simulink model "os_polyphase_synth_4096c"  (R2021a) (continued)
== system os_polyphase_synth_4096c/pfb_fir/pol1_in1_tap1 == (continued)
      | C+P>>17+(D-ACIN)*B
      | C+P>>17+(D-ACIN)*B+CARRYIN
      | C+P>>17+(D-ACIN)*BCIN
      | C+P>>17+(D-ACIN)*BCIN+CARRYIN
      | C+P>>17+(D-ACIN)+CARRYIN
      | C+P>>17+A
      | C+P>>17+A*A
      | C+P>>17+A*A+CARRYIN
      | C+P>>17+A*B
      | C+P>>17+A*B+CARRYIN
      | C+P>>17+A*BCIN
      | C+P>>17+A*BCIN+CARRYIN
      | C+P>>17+A+CARRYIN
      | C+P>>17+ACIN
      | C+P>>17+ACIN*ACIN
      | C+P>>17+ACIN*ACIN+CARRYIN
      | C+P>>17+ACIN*B
      | C+P>>17+ACIN*B+CARRYIN
      | C+P>>17+ACIN*BCIN
      | C+P>>17+ACIN*BCIN+CARRYIN
      | C+P>>17+ACIN+CARRYIN
      | C+P>>17+B*(A+D)
      | C+P>>17+B*(A+D)+CARRYIN
      | C+P>>17+B*(ACIN+D)
      | C+P>>17+B*(ACIN+D)+CARRYIN
      | C+P>>17+B*(D+A)
      | C+P>>17+B*(D+A)+CARRYIN
      | C+P>>17+B*(D+ACIN)
      | C+P>>17+B*(D+ACIN)+CARRYIN
      | C+P>>17+B*(D-A)
      | C+P>>17+B*(D-A)+CARRYIN
      | C+P>>17+B*(D-ACIN)
      | C+P>>17+B*(D-ACIN)+CARRYIN
      | C+P>>17+B*A
      | C+P>>17+B*A+CARRYIN
      | C+P>>17+B*ACIN
      | C+P>>17+B*ACIN+CARRYIN
      | C+P>>17+B*D
      | C+P>>17+B*D+CARRYIN
      | C+P>>17+BCIN*(A+D)
      | C+P>>17+BCIN*(A+D)+CARRYIN
      | C+P>>17+BCIN*(ACIN+D)
      | C+P>>17+BCIN*(ACIN+D)+CARRYIN
      | C+P>>17+BCIN*(D+A)
      | C+P>>17+BCIN*(D+A)+CARRYIN
      | C+P>>17+BCIN*(D+ACIN)
      | C+P>>17+BCIN*(D+ACIN)+CARRYIN
      | C+P>>17+BCIN*(D-A)
      | C+P>>17+BCIN*(D-A)+CARRYIN
      | C+P>>17+BCIN*(D-ACIN)
      | C+P>>17+BCIN*(D-ACIN)+CARRYIN
      | C+P>>17+BCIN*A
      | C+P>>17+BCIN*A+CARRYIN
      | C+P>>17+BCIN*ACIN
      | C+P>>17+BCIN*ACIN+CARRYIN
      | C+P>>17+BCIN*D
      | C+P>>17+BCIN*D+CARRYIN
      | C+P>>17+C
      | C+P>>17+C+CARRYIN
      | C+P>>17+C+CONCAT
      | C+P>>17+C+CONCAT+CARRYIN
      | C+P>>17+C+P
      | C+P>>17+C+P+CARRYIN
      | C+P>>17+CARRYIN
      | C+P>>17+CONCAT
      | C+P>>17+CONCAT+C
      | C+P>>17+CONCAT+C+CARRYIN
      | C+P>>17+CONCAT+CARRYIN
      | C+P>>17+CONCAT+P
      | C+P>>17+CONCAT+P+CARRYIN
      | C+P>>17+D
      | C+P>>17+D*B
      | C+P>>17+D*B+CARRYIN
      | C+P>>17+D*BCIN
      | C+P>>17+D*BCIN+CARRYIN
      | C+P>>17+D*D
      | C+P>>17+D*D+CARRYIN
      | C+P>>17+D+CARRYIN
      | C+P>>17+P
      | C+P>>17+P+C
      | C+P>>17+P+C+CARRYIN
      | C+P>>17+P+CARRYIN
      | C+P>>17+P+CONCAT
      | C+P>>17+P+CONCAT+CARRYIN
      | C+P>>17+P+P
      | C+P>>17+P+P+CARRYIN
      | C+PCIN
      | C+PCIN+(A+D)
      | C+PCIN+(A+D)*(A+D)
      | C+PCIN+(A+D)*(A+D)+CARRYIN
      | C+PCIN+(A+D)*B
      | C+PCIN+(A+D)*B+CARRYIN
      | C+PCIN+(A+D)*BCIN
      | C+PCIN+(A+D)*BCIN+CARRYIN
      | C+PCIN+(A+D)+CARRYIN
      | C+PCIN+(ACIN+D)
      | C+PCIN+(ACIN+D)*(ACIN+D)
      | C+PCIN+(ACIN+D)*(ACIN+D)+CARRYIN
      | C+PCIN+(ACIN+D)*B
      | C+PCIN+(ACIN+D)*B+CARRYIN
      | C+PCIN+(ACIN+D)*BCIN
      | C+PCIN+(ACIN+D)*BCIN+CARRYIN
      | C+PCIN+(ACIN+D)+CARRYIN
      | C+PCIN+(D+A)
      | C+PCIN+(D+A)*(D+A)
      | C+PCIN+(D+A)*(D+A)+CARRYIN
      | C+PCIN+(D+A)*B
      | C+PCIN+(D+A)*B+CARRYIN
      | C+PCIN+(D+A)*BCIN
      | C+PCIN+(D+A)*BCIN+CARRYIN
      | C+PCIN+(D+A)+CARRYIN
      | C+PCIN+(D+ACIN)
      | C+PCIN+(D+ACIN)*(D+ACIN)
      | C+PCIN+(D+ACIN)*(D+ACIN)+CARRYIN
      | C+PCIN+(D+ACIN)*B
      | C+PCIN+(D+ACIN)*B+CARRYIN
      | C+PCIN+(D+ACIN)*BCIN
      | C+PCIN+(D+ACIN)*BCIN+CARRYIN
      | C+PCIN+(D+ACIN)+CARRYIN
      | C+PCIN+(D-A)
      | C+PCIN+(D-A)*(D-A)
      | C+PCIN+(D-A)*(D-A)+CARRYIN
      | C+PCIN+(D-A)*B
      | C+PCIN+(D-A)*B+CARRYIN
      | C+PCIN+(D-A)*BCIN
      | C+PCIN+(D-A)*BCIN+CARRYIN
      | C+PCIN+(D-A)+CARRYIN
      | C+PCIN+(D-ACIN)
      | C+PCIN+(D-ACIN)*(D-ACIN)
      | C+PCIN+(D-ACIN)*(D-ACIN)+CARRYIN
      | C+PCIN+(D-ACIN)*B
      | C+PCIN+(D-ACIN)*B+CARRYIN
      | C+PCIN+(D-ACIN)*BCIN
      | C+PCIN+(D-ACIN)*BCIN+CARRYIN
      | C+PCIN+(D-ACIN)+CARRYIN
      | C+PCIN+A
      | C+PCIN+A*A
      | C+PCIN+A*A+CARRYIN
      | C+PCIN+A*B
      | C+PCIN+A*B+CARRYIN
      | C+PCIN+A*BCIN
      | C+PCIN+A*BCIN+CARRYIN
      | C+PCIN+A+CARRYIN
      | C+PCIN+ACIN
      | C+PCIN+ACIN*ACIN
      | C+PCIN+ACIN*ACIN+CARRYIN
      | C+PCIN+ACIN*B
      | C+PCIN+ACIN*B+CARRYIN
      | C+PCIN+ACIN*BCIN
      | C+PCIN+ACIN*BCIN+CARRYIN
      | C+PCIN+ACIN+CARRYIN
      | C+PCIN+B*(A+D)
      | C+PCIN+B*(A+D)+CARRYIN
      | C+PCIN+B*(ACIN+D)
      | C+PCIN+B*(ACIN+D)+CARRYIN
      | C+PCIN+B*(D+A)
      | C+PCIN+B*(D+A)+CARRYIN
      | C+PCIN+B*(D+ACIN)
      | C+PCIN+B*(D+ACIN)+CARRYIN
      | C+PCIN+B*(D-A)
      | C+PCIN+B*(D-A)+CARRYIN
      | C+PCIN+B*(D-ACIN)
      | C+PCIN+B*(D-ACIN)+CARRYIN
      | C+PCIN+B*A
      | C+PCIN+B*A+CARRYIN
      | C+PCIN+B*ACIN
      | C+PCIN+B*ACIN+CARRYIN
      | C+PCIN+B*D
      | C+PCIN+B*D+CARRYIN
      | C+PCIN+BCIN*(A+D)
      | C+PCIN+BCIN*(A+D)+CARRYIN
      | C+PCIN+BCIN*(ACIN+D)
      | C+PCIN+BCIN*(ACIN+D)+CARRYIN
      | C+PCIN+BCIN*(D+A)
      | C+PCIN+BCIN*(D+A)+CARRYIN
      | C+PCIN+BCIN*(D+ACIN)
      | C+PCIN+BCIN*(D+ACIN)+CARRYIN
      | C+PCIN+BCIN*(D-A)
      | C+PCIN+BCIN*(D-A)+CARRYIN
      | C+PCIN+BCIN*(D-ACIN)
      | C+PCIN+BCIN*(D-ACIN)+CARRYIN
      | C+PCIN+BCIN*A
      | C+PCIN+BCIN*A+CARRYIN
      | C+PCIN+BCIN*ACIN
      | C+PCIN+BCIN*ACIN+CARRYIN
      | C+PCIN+BCIN*D
      | C+PCIN+BCIN*D+CARRYIN
      | C+PCIN+C
      | C+PCIN+C+CARRYIN
      | C+PCIN+C+CONCAT
      | C+PCIN+C+CONCAT+CARRYIN
      | C+PCIN+C+P
      | C+PCIN+C+P+CARRYIN
      | C+PCIN+CARRYIN
      | C+PCIN+CONCAT
      | C+PCIN+CONCAT+C
      | C+PCIN+CONCAT+C+CARRYIN
      | C+PCIN+CONCAT+CARRYIN
      | C+PCIN+CONCAT+P
      | C+PCIN+CONCAT+P+CARRYIN
      | C+PCIN+D
      | C+PCIN+D*B
      | C+PCIN+D*B+CARRYIN
      | C+PCIN+D*BCIN
      | C+PCIN+D*BCIN+CARRYIN
      | C+PCIN+D*D
      | C+PCIN+D*D+CARRYIN
      | C+PCIN+D+CARRYIN
      | C+PCIN+P
      | C+PCIN+P+C
      | C+PCIN+P+C+CARRYIN
      | C+PCIN+P+CARRYIN
      | C+PCIN+P+CONCAT
      | C+PCIN+P+CONCAT+CARRYIN
      | C+PCIN+P+P
      | C+PCIN+P+P+CARRYIN
      | C+PCIN>>17
      | C+PCIN>>17+(A+D)
      | C+PCIN>>17+(A+D)*(A+D)
      | C+PCIN>>17+(A+D)*(A+D)+CARRYIN
      | C+PCIN>>17+(A+D)*B
      | C+PCIN>>17+(A+D)*B+CARRYIN
      | C+PCIN>>17+(A+D)*BCIN
      | C+PCIN>>17+(A+D)*BCIN+CARRYIN
      | C+PCIN>>17+(A+D)+CARRYIN
      | C+PCIN>>17+(ACIN+D)
      | C+PCIN>>17+(ACIN+D)*(ACIN+D)
      | C+PCIN>>17+(ACIN+D)*(ACIN+D)+CARRYIN
      | C+PCIN>>17+(ACIN+D)*B
      | C+PCIN>>17+(ACIN+D)*B+CARRYIN
      | C+PCIN>>17+(ACIN+D)*BCIN
      | C+PCIN>>17+(ACIN+D)*BCIN+CARRYIN
      | C+PCIN>>17+(ACIN+D)+CARRYIN
      | C+PCIN>>17+(D+A)
      | C+PCIN>>17+(D+A)*(D+A)
      | C+PCIN>>17+(D+A)*(D+A)+CARRYIN
      | C+PCIN>>17+(D+A)*B
      | C+PCIN>>17+(D+A)*B+CARRYIN
      | C+PCIN>>17+(D+A)*BCIN
      | C+PCIN>>17+(D+A)*BCIN+CARRYIN
      | C+PCIN>>17+(D+A)+CARRYIN
      | C+PCIN>>17+(D+ACIN)
      | C+PCIN>>17+(D+ACIN)*(D+ACIN)
      | C+PCIN>>17+(D+ACIN)*(D+ACIN)+CARRYIN
      | C+PCIN>>17+(D+ACIN)*B
      | C+PCIN>>17+(D+ACIN)*B+CARRYIN
      | C+PCIN>>17+(D+ACIN)*BCIN
      | C+PCIN>>17+(D+ACIN)*BCIN+CARRYIN
      | C+PCIN>>17+(D+ACIN)+CARRYIN
      | C+PCIN>>17+(D-A)
      | C+PCIN>>17+(D-A)*(D-A)
      | C+PCIN>>17+(D-A)*(D-A)+CARRYIN
      | C+PCIN>>17+(D-A)*B
      | C+PCIN>>17+(D-A)*B+CARRYIN
      | C+PCIN>>17+(D-A)*BCIN
      | C+PCIN>>17+(D-A)*BCIN+CARRYIN
      | C+PCIN>>17+(D-A)+CARRYIN
      | C+PCIN>>17+(D-ACIN)
      | C+PCIN>>17+(D-ACIN)*(D-ACIN)
      | C+PCIN>>17+(D-ACIN)*(D-ACIN)+CARRYIN
      | C+PCIN>>17+(D-ACIN)*B
      | C+PCIN>>17+(D-ACIN)*B+CARRYIN
      | C+PCIN>>17+(D-ACIN)*BCIN
      | C+PCIN>>17+(D-ACIN)*BCIN+CARRYIN
      | C+PCIN>>17+(D-ACIN)+CARRYIN
      | C+PCIN>>17+A
      | C+PCIN>>17+A*A
      | C+PCIN>>17+A*A+CARRYIN
      | C+PCIN>>17+A*B
      | C+PCIN>>17+A*B+CARRYIN
      | C+PCIN>>17+A*BCIN
      | C+PCIN>>17+A*BCIN+CARRYIN
      | C+PCIN>>17+A+CARRYIN
      | C+PCIN>>17+ACIN
      | C+PCIN>>17+ACIN*ACIN
      | C+PCIN>>17+ACIN*ACIN+CARRYIN
      | C+PCIN>>17+ACIN*B
      | C+PCIN>>17+ACIN*B+CARRYIN
      | C+PCIN>>17+ACIN*BCIN
      | C+PCIN>>17+ACIN*BCIN+CARRYIN
      | C+PCIN>>17+ACIN+CARRYIN
      | C+PCIN>>17+B*(A+D)
      | C+PCIN>>17+B*(A+D)+CARRYIN
      | C+PCIN>>17+B*(ACIN+D)
      | C+PCIN>>17+B*(ACIN+D)+CARRYIN
      | C+PCIN>>17+B*(D+A)
      | C+PCIN>>17+B*(D+A)+CARRYIN
      | C+PCIN>>17+B*(D+ACIN)
      | C+PCIN>>17+B*(D+ACIN)+CARRYIN
      | C+PCIN>>17+B*(D-A)
      | C+PCIN>>17+B*(D-A)+CARRYIN
      | C+PCIN>>17+B*(D-ACIN)
      | C+PCIN>>17+B*(D-ACIN)+CARRYIN
      | C+PCIN>>17+B*A
      | C+PCIN>>17+B*A+CARRYIN
      | C+PCIN>>17+B*ACIN
      | C+PCIN>>17+B*ACIN+CARRYIN
      | C+PCIN>>17+B*D
      | C+PCIN>>17+B*D+CARRYIN
      | C+PCIN>>17+BCIN*(A+D)
      | C+PCIN>>17+BCIN*(A+D)+CARRYIN
      | C+PCIN>>17+BCIN*(ACIN+D)
      | C+PCIN>>17+BCIN*(ACIN+D)+CARRYIN
      | C+PCIN>>17+BCIN*(D+A)
      | C+PCIN>>17+BCIN*(D+A)+CARRYIN
      | C+PCIN>>17+BCIN*(D+ACIN)
      | C+PCIN>>17+BCIN*(D+ACIN)+CARRYIN
      | C+PCIN>>17+BCIN*(D-A)
      | C+PCIN>>17+BCIN*(D-A)+CARRYIN
      | C+PCIN>>17+BCIN*(D-ACIN)
      | C+PCIN>>17+BCIN*(D-ACIN)+CARRYIN
      | C+PCIN>>17+BCIN*A
      | C+PCIN>>17+BCIN*A+CARRYIN
      | C+PCIN>>17+BCIN*ACIN
      | C+PCIN>>17+BCIN*ACIN+CARRYIN
      | C+PCIN>>17+BCIN*D
      | C+PCIN>>17+BCIN*D+CARRYIN
      | C+PCIN>>17+C
      | C+PCIN>>17+C+CARRYIN
      | C+PCIN>>17+C+CONCAT
      | C+PCIN>>17+C+CONCAT+CARRYIN
      | C+PCIN>>17+C+P
      | C+PCIN>>17+C+P+CARRYIN
      | C+PCIN>>17+CARRYIN
      | C+PCIN>>17+CONCAT
      | C+PCIN>>17+CONCAT+C
      | C+PCIN>>17+CONCAT+C+CARRYIN
      | C+PCIN>>17+CONCAT+CARRYIN
      | C+PCIN>>17+CONCAT+P
      | C+PCIN>>17+CONCAT+P+CARRYIN
      | C+PCIN>>17+D
      | C+PCIN>>17+D*B
      | C+PCIN>>17+D*B+CARRYIN
      | C+PCIN>>17+D*BCIN
      | C+PCIN>>17+D*BCIN+CARRYIN
      | C+PCIN>>17+D*D
      | C+PCIN>>17+D*D+CARRYIN
      | C+PCIN>>17+D+CARRYIN
      | C+PCIN>>17+P
      | C+PCIN>>17+P+C
      | C+PCIN>>17+P+C+CARRYIN
      | C+PCIN>>17+P+CARRYIN
      | C+PCIN>>17+P+CONCAT
      | C+PCIN>>17+P+CONCAT+CARRYIN
      | C+PCIN>>17+P+P
      | C+PCIN>>17+P+P+CARRYIN
      | C-((A+D))
      | C-((A+D)*(A+D))
      | C-((A+D)*(A+D)+C)
      | C-((A+D)*(A+D)+C+CARRYIN)
      | C-((A+D)*(A+D)+CARRYIN)
      | C-((A+D)*(A+D)+P)
      | C-((A+D)*(A+D)+P+CARRYIN)
      | C-((A+D)*B)
      | C-((A+D)*B+C)
      | C-((A+D)*B+C+CARRYIN)
      | C-((A+D)*B+CARRYIN)
      | C-((A+D)*B+P)
      | C-((A+D)*B+P+CARRYIN)
      | C-((A+D)*BCIN)
      | C-((A+D)*BCIN+C)
      | C-((A+D)*BCIN+C+CARRYIN)
      | C-((A+D)*BCIN+CARRYIN)
      | C-((A+D)*BCIN+P)
      | C-((A+D)*BCIN+P+CARRYIN)
      | C-((A+D)+C)
      | C-((A+D)+C+CARRYIN)
      | C-((A+D)+CARRYIN)
      | C-((A+D)+P)
      | C-((A+D)+P+CARRYIN)
      | C-((ACIN+D))
      | C-((ACIN+D)*(ACIN+D))
      | C-((ACIN+D)*(ACIN+D)+C)
      | C-((ACIN+D)*(ACIN+D)+C+CARRYIN)
      | C-((ACIN+D)*(ACIN+D)+CARRYIN)
      | C-((ACIN+D)*(ACIN+D)+P)
      | C-((ACIN+D)*(ACIN+D)+P+CARRYIN)
      | C-((ACIN+D)*B)
      | C-((ACIN+D)*B+C)
      | C-((ACIN+D)*B+C+CARRYIN)
      | C-((ACIN+D)*B+CARRYIN)
      | C-((ACIN+D)*B+P)
      | C-((ACIN+D)*B+P+CARRYIN)
      | C-((ACIN+D)*BCIN)
      | C-((ACIN+D)*BCIN+C)
      | C-((ACIN+D)*BCIN+C+CARRYIN)
      | C-((ACIN+D)*BCIN+CARRYIN)
      | C-((ACIN+D)*BCIN+P)
      | C-((ACIN+D)*BCIN+P+CARRYIN)
      | C-((ACIN+D)+C)
      | C-((ACIN+D)+C+CARRYIN)
      | C-((ACIN+D)+CARRYIN)
      | C-((ACIN+D)+P)
      | C-((ACIN+D)+P+CARRYIN)
      | C-((D+A))
      | C-((D+A)*(D+A))
      | C-((D+A)*(D+A)+C)
      | C-((D+A)*(D+A)+C+CARRYIN)
      | C-((D+A)*(D+A)+CARRYIN)
      | C-((D+A)*(D+A)+P)
      | C-((D+A)*(D+A)+P+CARRYIN)
      | C-((D+A)*B)
      | C-((D+A)*B+C)
      | C-((D+A)*B+C+CARRYIN)
      | C-((D+A)*B+CARRYIN)
      | C-((D+A)*B+P)
      | C-((D+A)*B+P+CARRYIN)
      | C-((D+A)*BCIN)
      | C-((D+A)*BCIN+C)
      | C-((D+A)*BCIN+C+CARRYIN)
      | C-((D+A)*BCIN+CARRYIN)
      | C-((D+A)*BCIN+P)
      | C-((D+A)*BCIN+P+CARRYIN)
      | C-((D+A)+C)
      | C-((D+A)+C+CARRYIN)
      | C-((D+A)+CARRYIN)
      | C-((D+A)+P)
      | C-((D+A)+P+CARRYIN)
      | C-((D+ACIN))
      | C-((D+ACIN)*(D+ACIN))
      | C-((D+ACIN)*(D+ACIN)+C)
      | C-((D+ACIN)*(D+ACIN)+C+CARRYIN)
      | C-((D+ACIN)*(D+ACIN)+CARRYIN)
      | C-((D+ACIN)*(D+ACIN)+P)
      | C-((D+ACIN)*(D+ACIN)+P+CARRYIN)
      | C-((D+ACIN)*B)
      | C-((D+ACIN)*B+C)
      | C-((D+ACIN)*B+C+CARRYIN)
      | C-((D+ACIN)*B+CARRYIN)
      | C-((D+ACIN)*B+P)
      | C-((D+ACIN)*B+P+CARRYIN)
      | C-((D+ACIN)*BCIN)
      | C-((D+ACIN)*BCIN+C)
      | C-((D+ACIN)*BCIN+C+CARRYIN)
      | C-((D+ACIN)*BCIN+CARRYIN)
      | C-((D+ACIN)*BCIN+P)
      | C-((D+ACIN)*BCIN+P+CARRYIN)
      | C-((D+ACIN)+C)
      | C-((D+ACIN)+C+CARRYIN)
      | C-((D+ACIN)+CARRYIN)
      | C-((D+ACIN)+P)
      | C-((D+ACIN)+P+CARRYIN)
      | C-((D-A))
      | C-((D-A)*(D-A))
      | C-((D-A)*(D-A)+C)
      | C-((D-A)*(D-A)+C+CARRYIN)
      | C-((D-A)*(D-A)+CARRYIN)
      | C-((D-A)*(D-A)+P)
      | C-((D-A)*(D-A)+P+CARRYIN)
      | C-((D-A)*B)
      | C-((D-A)*B+C)
      | C-((D-A)*B+C+CARRYIN)
      | C-((D-A)*B+CARRYIN)
      | C-((D-A)*B+P)
      | C-((D-A)*B+P+CARRYIN)
      | C-((D-A)*BCIN)
      | C-((D-A)*BCIN+C)
      | C-((D-A)*BCIN+C+CARRYIN)
      | C-((D-A)*BCIN+CARRYIN)
      | C-((D-A)*BCIN+P)
      | C-((D-A)*BCIN+P+CARRYIN)
      | C-((D-A)+C)
      | C-((D-A)+C+CARRYIN)
      | C-((D-A)+CARRYIN)
      | C-((D-A)+P)
      | C-((D-A)+P+CARRYIN)
      | C-((D-ACIN))
      | C-((D-ACIN)*(D-ACIN))
      | C-((D-ACIN)*(D-ACIN)+C)
      | C-((D-ACIN)*(D-ACIN)+C+CARRYIN)
      | C-((D-ACIN)*(D-ACIN)+CARRYIN)
      | C-((D-ACIN)*(D-ACIN)+P)
      | C-((D-ACIN)*(D-ACIN)+P+CARRYIN)
      | C-((D-ACIN)*B)
      | C-((D-ACIN)*B+C)
      | C-((D-ACIN)*B+C+CARRYIN)
      | C-((D-ACIN)*B+CARRYIN)
      | C-((D-ACIN)*B+P)
      | C-((D-ACIN)*B+P+CARRYIN)
      | C-((D-ACIN)*BCIN)
      | C-((D-ACIN)*BCIN+C)
      | C-((D-ACIN)*BCIN+C+CARRYIN)
      | C-((D-ACIN)*BCIN+CARRYIN)
      | C-((D-ACIN)*BCIN+P)
      | C-((D-ACIN)*BCIN+P+CARRYIN)
      | C-((D-ACIN)+C)
      | C-((D-ACIN)+C+CARRYIN)
      | C-((D-ACIN)+CARRYIN)
      | C-((D-ACIN)+P)
      | C-((D-ACIN)+P+CARRYIN)
      | C-(A)
      | C-(A*A)
      | C-(A*A+C)
      | C-(A*A+C+CARRYIN)
      | C-(A*A+CARRYIN)
      | C-(A*A+P)
      | C-(A*A+P+CARRYIN)
      | C-(A*B)
      | C-(A*B+C)
      | C-(A*B+C+CARRYIN)
      | C-(A*B+CARRYIN)
      | C-(A*B+P)
      | C-(A*B+P+CARRYIN)
      | C-(A*BCIN)
      | C-(A*BCIN+C)
      | C-(A*BCIN+C+CARRYIN)
      | C-(A*BCIN+CARRYIN)
      | C-(A*BCIN+P)
      | C-(A*BCIN+P+CARRYIN)
      | C-(A+C)
      | C-(A+C+CARRYIN)
      | C-(A+CARRYIN)
      | C-(A+D)
      | C-(A+D)*(A+D)
      | C-(A+D)*(A+D)-C
      | C-(A+D)*(A+D)-C-CARRYIN
      | C-(A+D)*(A+D)-CARRYIN
      | C-(A+D)*(A+D)-P
      | C-(A+D)*(A+D)-P-CARRYIN
      | C-(A+D)*B
      | C-(A+D)*B-C
      | C-(A+D)*B-C-CARRYIN
      | C-(A+D)*B-CARRYIN
      | C-(A+D)*B-P
      | C-(A+D)*B-P-CARRYIN
      | C-(A+D)*BCIN
      | C-(A+D)*BCIN-C
      | C-(A+D)*BCIN-C-CARRYIN
      | C-(A+D)*BCIN-CARRYIN
      | C-(A+D)*BCIN-P
      | C-(A+D)*BCIN-P-CARRYIN
      | C-(A+D)-C
      | C-(A+D)-C-CARRYIN
      | C-(A+D)-CARRYIN
      | C-(A+D)-P
      | C-(A+D)-P-CARRYIN
      | C-(A+P)
      | C-(A+P+CARRYIN)
      | C-(ACIN)
      | C-(ACIN*ACIN)
      | C-(ACIN*ACIN+C)
      | C-(ACIN*ACIN+C+CARRYIN)
      | C-(ACIN*ACIN+CARRYIN)
      | C-(ACIN*ACIN+P)
      | C-(ACIN*ACIN+P+CARRYIN)
      | C-(ACIN*B)
      | C-(ACIN*B+C)
      | C-(ACIN*B+C+CARRYIN)
      | C-(ACIN*B+CARRYIN)
      | C-(ACIN*B+P)
      | C-(ACIN*B+P+CARRYIN)
      | C-(ACIN*BCIN)
      | C-(ACIN*BCIN+C)
      | C-(ACIN*BCIN+C+CARRYIN)
      | C-(ACIN*BCIN+CARRYIN)
      | C-(ACIN*BCIN+P)
      | C-(ACIN*BCIN+P+CARRYIN)
      | C-(ACIN+C)
      | C-(ACIN+C+CARRYIN)
      | C-(ACIN+CARRYIN)
      | C-(ACIN+D)
      | C-(ACIN+D)*(ACIN+D)
      | C-(ACIN+D)*(ACIN+D)-C
      | C-(ACIN+D)*(ACIN+D)-C-CARRYIN
      | C-(ACIN+D)*(ACIN+D)-CARRYIN
      | C-(ACIN+D)*(ACIN+D)-P
      | C-(ACIN+D)*(ACIN+D)-P-CARRYIN
      | C-(ACIN+D)*B
      | C-(ACIN+D)*B-C
      | C-(ACIN+D)*B-C-CARRYIN
      | C-(ACIN+D)*B-CARRYIN
      | C-(ACIN+D)*B-P
      | C-(ACIN+D)*B-P-CARRYIN
      | C-(ACIN+D)*BCIN
      | C-(ACIN+D)*BCIN-C
      | C-(ACIN+D)*BCIN-C-CARRYIN
      | C-(ACIN+D)*BCIN-CARRYIN
      | C-(ACIN+D)*BCIN-P
      | C-(ACIN+D)*BCIN-P-CARRYIN
      | C-(ACIN+D)-C
      | C-(ACIN+D)-C-CARRYIN
      | C-(ACIN+D)-CARRYIN
      | C-(ACIN+D)-P
      | C-(ACIN+D)-P-CARRYIN
      | C-(ACIN+P)
      | C-(ACIN+P+CARRYIN)
      | C-(B*(A+D))
      | C-(B*(A+D)+C)
      | C-(B*(A+D)+C+CARRYIN)
      | C-(B*(A+D)+CARRYIN)
      | C-(B*(A+D)+P)
      | C-(B*(A+D)+P+CARRYIN)
      | C-(B*(ACIN+D))
      | C-(B*(ACIN+D)+C)
      | C-(B*(ACIN+D)+C+CARRYIN)
      | C-(B*(ACIN+D)+CARRYIN)
      | C-(B*(ACIN+D)+P)
      | C-(B*(ACIN+D)+P+CARRYIN)
      | C-(B*(D+A))
      | C-(B*(D+A)+C)
      | C-(B*(D+A)+C+CARRYIN)
      | C-(B*(D+A)+CARRYIN)
      | C-(B*(D+A)+P)
      | C-(B*(D+A)+P+CARRYIN)
      | C-(B*(D+ACIN))
      | C-(B*(D+ACIN)+C)
      | C-(B*(D+ACIN)+C+CARRYIN)
      | C-(B*(D+ACIN)+CARRYIN)
      | C-(B*(D+ACIN)+P)
      | C-(B*(D+ACIN)+P+CARRYIN)
      | C-(B*(D-A))
      | C-(B*(D-A)+C)
      | C-(B*(D-A)+C+CARRYIN)
      | C-(B*(D-A)+CARRYIN)
      | C-(B*(D-A)+P)
      | C-(B*(D-A)+P+CARRYIN)
      | C-(B*(D-ACIN))
      | C-(B*(D-ACIN)+C)
      | C-(B*(D-ACIN)+C+CARRYIN)
      | C-(B*(D-ACIN)+CARRYIN)
      | C-(B*(D-ACIN)+P)
      | C-(B*(D-ACIN)+P+CARRYIN)
      | C-(B*A)
      | C-(B*A+C)
      | C-(B*A+C+CARRYIN)
      | C-(B*A+CARRYIN)
      | C-(B*A+P)
      | C-(B*A+P+CARRYIN)
      | C-(B*ACIN)
      | C-(B*ACIN+C)
      | C-(B*ACIN+C+CARRYIN)
      | C-(B*ACIN+CARRYIN)
      | C-(B*ACIN+P)
      | C-(B*ACIN+P+CARRYIN)
      | C-(B*D)
      | C-(B*D+C)
      | C-(B*D+C+CARRYIN)
      | C-(B*D+CARRYIN)
      | C-(B*D+P)
      | C-(B*D+P+CARRYIN)
      | C-(BCIN*(A+D))
      | C-(BCIN*(A+D)+C)
      | C-(BCIN*(A+D)+C+CARRYIN)
      | C-(BCIN*(A+D)+CARRYIN)
      | C-(BCIN*(A+D)+P)
      | C-(BCIN*(A+D)+P+CARRYIN)
      | C-(BCIN*(ACIN+D))
      | C-(BCIN*(ACIN+D)+C)
      | C-(BCIN*(ACIN+D)+C+CARRYIN)
      | C-(BCIN*(ACIN+D)+CARRYIN)
      | C-(BCIN*(ACIN+D)+P)
      | C-(BCIN*(ACIN+D)+P+CARRYIN)
      | C-(BCIN*(D+A))
      | C-(BCIN*(D+A)+C)
      | C-(BCIN*(D+A)+C+CARRYIN)
      | C-(BCIN*(D+A)+CARRYIN)
      | C-(BCIN*(D+A)+P)
      | C-(BCIN*(D+A)+P+CARRYIN)
      | C-(BCIN*(D+ACIN))
      | C-(BCIN*(D+ACIN)+C)
      | C-(BCIN*(D+ACIN)+C+CARRYIN)
      | C-(BCIN*(D+ACIN)+CARRYIN)
      | C-(BCIN*(D+ACIN)+P)
      | C-(BCIN*(D+ACIN)+P+CARRYIN)
      | C-(BCIN*(D-A))
      | C-(BCIN*(D-A)+C)
      | C-(BCIN*(D-A)+C+CARRYIN)
      | C-(BCIN*(D-A)+CARRYIN)
      | C-(BCIN*(D-A)+P)
      | C-(BCIN*(D-A)+P+CARRYIN)
      | C-(BCIN*(D-ACIN))
      | C-(BCIN*(D-ACIN)+C)
      | C-(BCIN*(D-ACIN)+C+CARRYIN)
      | C-(BCIN*(D-ACIN)+CARRYIN)
      | C-(BCIN*(D-ACIN)+P)
      | C-(BCIN*(D-ACIN)+P+CARRYIN)
      | C-(BCIN*A)
      | C-(BCIN*A+C)
      | C-(BCIN*A+C+CARRYIN)
      | C-(BCIN*A+CARRYIN)
      | C-(BCIN*A+P)
      | C-(BCIN*A+P+CARRYIN)
      | C-(BCIN*ACIN)
      | C-(BCIN*ACIN+C)
      | C-(BCIN*ACIN+C+CARRYIN)
      | C-(BCIN*ACIN+CARRYIN)
      | C-(BCIN*ACIN+P)
      | C-(BCIN*ACIN+P+CARRYIN)
      | C-(BCIN*D)
      | C-(BCIN*D+C)
      | C-(BCIN*D+C+CARRYIN)
      | C-(BCIN*D+CARRYIN)
      | C-(BCIN*D+P)
      | C-(BCIN*D+P+CARRYIN)
      | C-(C)
      | C-(C+(A+D))
      | C-(C+(A+D)*(A+D))
      | C-(C+(A+D)*(A+D)+CARRYIN)
      | C-(C+(A+D)*B)
      | C-(C+(A+D)*B+CARRYIN)
      | C-(C+(A+D)*BCIN)
      | C-(C+(A+D)*BCIN+CARRYIN)
      | C-(C+(A+D)+CARRYIN)
      | C-(C+(ACIN+D))
      | C-(C+(ACIN+D)*(ACIN+D))
      | C-(C+(ACIN+D)*(ACIN+D)+CARRYIN)
      | C-(C+(ACIN+D)*B)
      | C-(C+(ACIN+D)*B+CARRYIN)
      | C-(C+(ACIN+D)*BCIN)
      | C-(C+(ACIN+D)*BCIN+CARRYIN)
      | C-(C+(ACIN+D)+CARRYIN)
      | C-(C+(D+A))
      | C-(C+(D+A)*(D+A))
      | C-(C+(D+A)*(D+A)+CARRYIN)
      | C-(C+(D+A)*B)
      | C-(C+(D+A)*B+CARRYIN)
      | C-(C+(D+A)*BCIN)
      | C-(C+(D+A)*BCIN+CARRYIN)
      | C-(C+(D+A)+CARRYIN)
      | C-(C+(D+ACIN))
      | C-(C+(D+ACIN)*(D+ACIN))
      | C-(C+(D+ACIN)*(D+ACIN)+CARRYIN)
      | C-(C+(D+ACIN)*B)
      | C-(C+(D+ACIN)*B+CARRYIN)
      | C-(C+(D+ACIN)*BCIN)
      | C-(C+(D+ACIN)*BCIN+CARRYIN)
      | C-(C+(D+ACIN)+CARRYIN)
      | C-(C+(D-A))
      | C-(C+(D-A)*(D-A))
      | C-(C+(D-A)*(D-A)+CARRYIN)
      | C-(C+(D-A)*B)
      | C-(C+(D-A)*B+CARRYIN)
      | C-(C+(D-A)*BCIN)
      | C-(C+(D-A)*BCIN+CARRYIN)
      | C-(C+(D-A)+CARRYIN)
      | C-(C+(D-ACIN))
      | C-(C+(D-ACIN)*(D-ACIN))
      | C-(C+(D-ACIN)*(D-ACIN)+CARRYIN)
      | C-(C+(D-ACIN)*B)
      | C-(C+(D-ACIN)*B+CARRYIN)
      | C-(C+(D-ACIN)*BCIN)
      | C-(C+(D-ACIN)*BCIN+CARRYIN)
      | C-(C+(D-ACIN)+CARRYIN)
      | C-(C+A)
      | C-(C+A*A)
      | C-(C+A*A+CARRYIN)
      | C-(C+A*B)
      | C-(C+A*B+CARRYIN)
      | C-(C+A*BCIN)
      | C-(C+A*BCIN+CARRYIN)
      | C-(C+A+CARRYIN)
      | C-(C+ACIN)
      | C-(C+ACIN*ACIN)
      | C-(C+ACIN*ACIN+CARRYIN)
      | C-(C+ACIN*B)
      | C-(C+ACIN*B+CARRYIN)
      | C-(C+ACIN*BCIN)
      | C-(C+ACIN*BCIN+CARRYIN)
      | C-(C+ACIN+CARRYIN)
      | C-(C+B*(A+D))
      | C-(C+B*(A+D)+CARRYIN)
      | C-(C+B*(ACIN+D))
      | C-(C+B*(ACIN+D)+CARRYIN)
      | C-(C+B*(D+A))
      | C-(C+B*(D+A)+CARRYIN)
      | C-(C+B*(D+ACIN))
      | C-(C+B*(D+ACIN)+CARRYIN)
      | C-(C+B*(D-A))
      | C-(C+B*(D-A)+CARRYIN)
      | C-(C+B*(D-ACIN))
      | C-(C+B*(D-ACIN)+CARRYIN)
      | C-(C+B*A)
      | C-(C+B*A+CARRYIN)
      | C-(C+B*ACIN)
      | C-(C+B*ACIN+CARRYIN)
      | C-(C+B*D)
      | C-(C+B*D+CARRYIN)
      | C-(C+BCIN*(A+D))
      | C-(C+BCIN*(A+D)+CARRYIN)
      | C-(C+BCIN*(ACIN+D))
      | C-(C+BCIN*(ACIN+D)+CARRYIN)
      | C-(C+BCIN*(D+A))
      | C-(C+BCIN*(D+A)+CARRYIN)
      | C-(C+BCIN*(D+ACIN))
      | C-(C+BCIN*(D+ACIN)+CARRYIN)
      | C-(C+BCIN*(D-A))
      | C-(C+BCIN*(D-A)+CARRYIN)
      | C-(C+BCIN*(D-ACIN))
      | C-(C+BCIN*(D-ACIN)+CARRYIN)
      | C-(C+BCIN*A)
      | C-(C+BCIN*A+CARRYIN)
      | C-(C+BCIN*ACIN)
      | C-(C+BCIN*ACIN+CARRYIN)
      | C-(C+BCIN*D)
      | C-(C+BCIN*D+CARRYIN)
      | C-(C+C)
      | C-(C+C+CARRYIN)
      | C-(C+C+CONCAT)
      | C-(C+C+CONCAT+CARRYIN)
      | C-(C+C+P)
      | C-(C+C+P+CARRYIN)
      | C-(C+CARRYCASCIN)
      | C-(C+CARRYIN)
      | C-(C+CONCAT)
      | C-(C+CONCAT+C)
      | C-(C+CONCAT+C+CARRYIN)
      | C-(C+CONCAT+CARRYIN)
      | C-(C+CONCAT+P)
      | C-(C+CONCAT+P+CARRYIN)
      | C-(C+D)
      | C-(C+D*B)
      | C-(C+D*B+CARRYIN)
      | C-(C+D*BCIN)
      | C-(C+D*BCIN+CARRYIN)
      | C-(C+D*D)
      | C-(C+D*D+CARRYIN)
      | C-(C+D+CARRYIN)
      | C-(C+P)
      | C-(C+P+C)
      | C-(C+P+C+CARRYIN)
      | C-(C+P+CARRYIN)
      | C-(C+P+CONCAT)
      | C-(C+P+CONCAT+CARRYIN)
      | C-(C+P+P)
      | C-(C+P+P+CARRYIN)
      | C-(CARRYCASCIN)
      | C-(CARRYIN)
      | C-(CONCAT)
      | C-(CONCAT+C)
      | C-(CONCAT+C+C)
      | C-(CONCAT+C+C+CARRYIN)
      | C-(CONCAT+C+CARRYIN)
      | C-(CONCAT+C+P)
      | C-(CONCAT+C+P+CARRYIN)
      | C-(CONCAT+CARRYCASCIN)
      | C-(CONCAT+CARRYIN)
      | C-(CONCAT+P)
      | C-(CONCAT+P+C)
      | C-(CONCAT+P+C+CARRYIN)
      | C-(CONCAT+P+CARRYIN)
      | C-(D)
      | C-(D*B)
      | C-(D*B+C)
      | C-(D*B+C+CARRYIN)
      | C-(D*B+CARRYIN)
      | C-(D*B+P)
      | C-(D*B+P+CARRYIN)
      | C-(D*BCIN)
      | C-(D*BCIN+C)
      | C-(D*BCIN+C+CARRYIN)
      | C-(D*BCIN+CARRYIN)
      | C-(D*BCIN+P)
      | C-(D*BCIN+P+CARRYIN)
      | C-(D*D)
      | C-(D*D+C)
      | C-(D*D+C+CARRYIN)
      | C-(D*D+CARRYIN)
      | C-(D*D+P)
      | C-(D*D+P+CARRYIN)
      | C-(D+A)
      | C-(D+A)*(D+A)
      | C-(D+A)*(D+A)-C
      | C-(D+A)*(D+A)-C-CARRYIN
      | C-(D+A)*(D+A)-CARRYIN
      | C-(D+A)*(D+A)-P
      | C-(D+A)*(D+A)-P-CARRYIN
      | C-(D+A)*B
      | C-(D+A)*B-C
      | C-(D+A)*B-C-CARRYIN
      | C-(D+A)*B-CARRYIN
      | C-(D+A)*B-P
      | C-(D+A)*B-P-CARRYIN
      | C-(D+A)*BCIN
      | C-(D+A)*BCIN-C
      | C-(D+A)*BCIN-C-CARRYIN
      | C-(D+A)*BCIN-CARRYIN
      | C-(D+A)*BCIN-P
      | C-(D+A)*BCIN-P-CARRYIN
      | C-(D+A)-C
      | C-(D+A)-C-CARRYIN
      | C-(D+A)-CARRYIN
      | C-(D+A)-P
      | C-(D+A)-P-CARRYIN
      | C-(D+ACIN)
      | C-(D+ACIN)*(D+ACIN)
      | C-(D+ACIN)*(D+ACIN)-C
      | C-(D+ACIN)*(D+ACIN)-C-CARRYIN
      | C-(D+ACIN)*(D+ACIN)-CARRYIN
      | C-(D+ACIN)*(D+ACIN)-P
      | C-(D+ACIN)*(D+ACIN)-P-CARRYIN
      | C-(D+ACIN)*B
      | C-(D+ACIN)*B-C
      | C-(D+ACIN)*B-C-CARRYIN
      | C-(D+ACIN)*B-CARRYIN
      | C-(D+ACIN)*B-P
      | C-(D+ACIN)*B-P-CARRYIN
      | C-(D+ACIN)*BCIN
      | C-(D+ACIN)*BCIN-C
      | C-(D+ACIN)*BCIN-C-CARRYIN
      | C-(D+ACIN)*BCIN-CARRYIN
      | C-(D+ACIN)*BCIN-P
      | C-(D+ACIN)*BCIN-P-CARRYIN
      | C-(D+ACIN)-C
      | C-(D+ACIN)-C-CARRYIN
      | C-(D+ACIN)-CARRYIN
      | C-(D+ACIN)-P
      | C-(D+ACIN)-P-CARRYIN
      | C-(D+C)
      | C-(D+C+CARRYIN)
      | C-(D+CARRYIN)
      | C-(D+P)
      | C-(D+P+CARRYIN)
      | C-(D-A)
      | C-(D-A)*(D-A)
      | C-(D-A)*(D-A)-C
      | C-(D-A)*(D-A)-C-CARRYIN
      | C-(D-A)*(D-A)-CARRYIN
      | C-(D-A)*(D-A)-P
      | C-(D-A)*(D-A)-P-CARRYIN
      | C-(D-A)*B
      | C-(D-A)*B-C
      | C-(D-A)*B-C-CARRYIN
      | C-(D-A)*B-CARRYIN
      | C-(D-A)*B-P
      | C-(D-A)*B-P-CARRYIN
      | C-(D-A)*BCIN
      | C-(D-A)*BCIN-C
      | C-(D-A)*BCIN-C-CARRYIN
      | C-(D-A)*BCIN-CARRYIN
      | C-(D-A)*BCIN-P
      | C-(D-A)*BCIN-P-CARRYIN
      | C-(D-A)-C
      | C-(D-A)-C-CARRYIN
      | C-(D-A)-CARRYIN
      | C-(D-A)-P
      | C-(D-A)-P-CARRYIN
      | C-(D-ACIN)
      | C-(D-ACIN)*(D-ACIN)
      | C-(D-ACIN)*(D-ACIN)-C
      | C-(D-ACIN)*(D-ACIN)-C-CARRYIN
      | C-(D-ACIN)*(D-ACIN)-CARRYIN
      | C-(D-ACIN)*(D-ACIN)-P
      | C-(D-ACIN)*(D-ACIN)-P-CARRYIN
      | C-(D-ACIN)*B
      | C-(D-ACIN)*B-C
      | C-(D-ACIN)*B-C-CARRYIN
      | C-(D-ACIN)*B-CARRYIN
      | C-(D-ACIN)*B-P
      | C-(D-ACIN)*B-P-CARRYIN
      | C-(D-ACIN)*BCIN
      | C-(D-ACIN)*BCIN-C
      | C-(D-ACIN)*BCIN-C-CARRYIN
      | C-(D-ACIN)*BCIN-CARRYIN
      | C-(D-ACIN)*BCIN-P
      | C-(D-ACIN)*BCIN-P-CARRYIN
      | C-(D-ACIN)-C
      | C-(D-ACIN)-C-CARRYIN
      | C-(D-ACIN)-CARRYIN
      | C-(D-ACIN)-P
      | C-(D-ACIN)-P-CARRYIN
      | C-(P)
      | C-(P+(A+D))
      | C-(P+(A+D)*(A+D))
      | C-(P+(A+D)*(A+D)+CARRYIN)
      | C-(P+(A+D)*B)
      | C-(P+(A+D)*B+CARRYIN)
      | C-(P+(A+D)*BCIN)
      | C-(P+(A+D)*BCIN+CARRYIN)
      | C-(P+(A+D)+CARRYIN)
      | C-(P+(ACIN+D))
      | C-(P+(ACIN+D)*(ACIN+D))
      | C-(P+(ACIN+D)*(ACIN+D)+CARRYIN)
      | C-(P+(ACIN+D)*B)
      | C-(P+(ACIN+D)*B+CARRYIN)
      | C-(P+(ACIN+D)*BCIN)
      | C-(P+(ACIN+D)*BCIN+CARRYIN)
      | C-(P+(ACIN+D)+CARRYIN)
      | C-(P+(D+A))
      | C-(P+(D+A)*(D+A))
      | C-(P+(D+A)*(D+A)+CARRYIN)
      | C-(P+(D+A)*B)
      | C-(P+(D+A)*B+CARRYIN)
      | C-(P+(D+A)*BCIN)
      | C-(P+(D+A)*BCIN+CARRYIN)
      | C-(P+(D+A)+CARRYIN)
      | C-(P+(D+ACIN))
      | C-(P+(D+ACIN)*(D+ACIN))
      | C-(P+(D+ACIN)*(D+ACIN)+CARRYIN)
      | C-(P+(D+ACIN)*B)
      | C-(P+(D+ACIN)*B+CARRYIN)
      | C-(P+(D+ACIN)*BCIN)
      | C-(P+(D+ACIN)*BCIN+CARRYIN)
      | C-(P+(D+ACIN)+CARRYIN)
      | C-(P+(D-A))
      | C-(P+(D-A)*(D-A))
      | C-(P+(D-A)*(D-A)+CARRYIN)
      | C-(P+(D-A)*B)
      | C-(P+(D-A)*B+CARRYIN)
      | C-(P+(D-A)*BCIN)
      | C-(P+(D-A)*BCIN+CARRYIN)
      | C-(P+(D-A)+CARRYIN)
      | C-(P+(D-ACIN))
      | C-(P+(D-ACIN)*(D-ACIN))
      | C-(P+(D-ACIN)*(D-ACIN)+CARRYIN)
      | C-(P+(D-ACIN)*B)
      | C-(P+(D-ACIN)*B+CARRYIN)
      | C-(P+(D-ACIN)*BCIN)
      | C-(P+(D-ACIN)*BCIN+CARRYIN)
      | C-(P+(D-ACIN)+CARRYIN)
      | C-(P+A)
      | C-(P+A*A)
      | C-(P+A*A+CARRYIN)
      | C-(P+A*B)
      | C-(P+A*B+CARRYIN)
      | C-(P+A*BCIN)
      | C-(P+A*BCIN+CARRYIN)
      | C-(P+A+CARRYIN)
      | C-(P+ACIN)
      | C-(P+ACIN*ACIN)
      | C-(P+ACIN*ACIN+CARRYIN)
      | C-(P+ACIN*B)
      | C-(P+ACIN*B+CARRYIN)
      | C-(P+ACIN*BCIN)
      | C-(P+ACIN*BCIN+CARRYIN)
      | C-(P+ACIN+CARRYIN)
      | C-(P+B*(A+D))
      | C-(P+B*(A+D)+CARRYIN)
      | C-(P+B*(ACIN+D))
      | C-(P+B*(ACIN+D)+CARRYIN)
      | C-(P+B*(D+A))
      | C-(P+B*(D+A)+CARRYIN)
      | C-(P+B*(D+ACIN))
      | C-(P+B*(D+ACIN)+CARRYIN)
      | C-(P+B*(D-A))
      | C-(P+B*(D-A)+CARRYIN)
      | C-(P+B*(D-ACIN))
      | C-(P+B*(D-ACIN)+CARRYIN)
      | C-(P+B*A)
      | C-(P+B*A+CARRYIN)
      | C-(P+B*ACIN)
      | C-(P+B*ACIN+CARRYIN)
      | C-(P+B*D)
      | C-(P+B*D+CARRYIN)
      | C-(P+BCIN*(A+D))
      | C-(P+BCIN*(A+D)+CARRYIN)
      | C-(P+BCIN*(ACIN+D))
      | C-(P+BCIN*(ACIN+D)+CARRYIN)
      | C-(P+BCIN*(D+A))
      | C-(P+BCIN*(D+A)+CARRYIN)
      | C-(P+BCIN*(D+ACIN))
      | C-(P+BCIN*(D+ACIN)+CARRYIN)
      | C-(P+BCIN*(D-A))
      | C-(P+BCIN*(D-A)+CARRYIN)
      | C-(P+BCIN*(D-ACIN))
      | C-(P+BCIN*(D-ACIN)+CARRYIN)
      | C-(P+BCIN*A)
      | C-(P+BCIN*A+CARRYIN)
      | C-(P+BCIN*ACIN)
      | C-(P+BCIN*ACIN+CARRYIN)
      | C-(P+BCIN*D)
      | C-(P+BCIN*D+CARRYIN)
      | C-(P+C)
      | C-(P+C+C)
      | C-(P+C+C+CARRYIN)
      | C-(P+C+CARRYIN)
      | C-(P+C+CONCAT)
      | C-(P+C+CONCAT+CARRYIN)
      | C-(P+C+P)
      | C-(P+C+P+CARRYIN)
      | C-(P+CARRYCASCIN)
      | C-(P+CARRYIN)
      | C-(P+CONCAT)
      | C-(P+CONCAT+C)
      | C-(P+CONCAT+C+CARRYIN)
      | C-(P+CONCAT+CARRYIN)
      | C-(P+D)
      | C-(P+D*B)
      | C-(P+D*B+CARRYIN)
      | C-(P+D*BCIN)
      | C-(P+D*BCIN+CARRYIN)
      | C-(P+D*D)
      | C-(P+D*D+CARRYIN)
      | C-(P+D+CARRYIN)
      | C-(P+P)
      | C-(P+P+C)
      | C-(P+P+C+CARRYIN)
      | C-(P+P+CARRYIN)
      | C-A
      | C-A*A
      | C-A*A-C
      | C-A*A-C-CARRYIN
      | C-A*A-CARRYIN
      | C-A*A-P
      | C-A*A-P-CARRYIN
      | C-A*B
      | C-A*B-C
      | C-A*B-C-CARRYIN
      | C-A*B-CARRYIN
      | C-A*B-P
      | C-A*B-P-CARRYIN
      | C-A*BCIN
      | C-A*BCIN-C
      | C-A*BCIN-C-CARRYIN
      | C-A*BCIN-CARRYIN
      | C-A*BCIN-P
      | C-A*BCIN-P-CARRYIN
      | C-A-C
      | C-A-C-CARRYIN
      | C-A-CARRYIN
      | C-A-P
      | C-A-P-CARRYIN
      | C-ACIN
      | C-ACIN*ACIN
      | C-ACIN*ACIN-C
      | C-ACIN*ACIN-C-CARRYIN
      | C-ACIN*ACIN-CARRYIN
      | C-ACIN*ACIN-P
      | C-ACIN*ACIN-P-CARRYIN
      | C-ACIN*B
      | C-ACIN*B-C
      | C-ACIN*B-C-CARRYIN
      | C-ACIN*B-CARRYIN
      | C-ACIN*B-P
      | C-ACIN*B-P-CARRYIN
      | C-ACIN*BCIN
      | C-ACIN*BCIN-C
      | C-ACIN*BCIN-C-CARRYIN
      | C-ACIN*BCIN-CARRYIN
      | C-ACIN*BCIN-P
      | C-ACIN*BCIN-P-CARRYIN
      | C-ACIN-C
      | C-ACIN-C-CARRYIN
      | C-ACIN-CARRYIN
      | C-ACIN-P
      | C-ACIN-P-CARRYIN
      | C-B*(A+D)
      | C-B*(A+D)-C
      | C-B*(A+D)-C-CARRYIN
      | C-B*(A+D)-CARRYIN
      | C-B*(A+D)-P
      | C-B*(A+D)-P-CARRYIN
      | C-B*(ACIN+D)
      | C-B*(ACIN+D)-C
      | C-B*(ACIN+D)-C-CARRYIN
      | C-B*(ACIN+D)-CARRYIN
      | C-B*(ACIN+D)-P
      | C-B*(ACIN+D)-P-CARRYIN
      | C-B*(D+A)
      | C-B*(D+A)-C
      | C-B*(D+A)-C-CARRYIN
      | C-B*(D+A)-CARRYIN
      | C-B*(D+A)-P
      | C-B*(D+A)-P-CARRYIN
      | C-B*(D+ACIN)
      | C-B*(D+ACIN)-C
      | C-B*(D+ACIN)-C-CARRYIN
      | C-B*(D+ACIN)-CARRYIN
      | C-B*(D+ACIN)-P
      | C-B*(D+ACIN)-P-CARRYIN
      | C-B*(D-A)
      | C-B*(D-A)-C
      | C-B*(D-A)-C-CARRYIN
      | C-B*(D-A)-CARRYIN
      | C-B*(D-A)-P
      | C-B*(D-A)-P-CARRYIN
      | C-B*(D-ACIN)
      | C-B*(D-ACIN)-C
      | C-B*(D-ACIN)-C-CARRYIN
      | C-B*(D-ACIN)-CARRYIN
      | C-B*(D-ACIN)-P
      | C-B*(D-ACIN)-P-CARRYIN
      | C-B*A
      | C-B*A-C
      | C-B*A-C-CARRYIN
      | C-B*A-CARRYIN
      | C-B*A-P
      | C-B*A-P-CARRYIN
      | C-B*ACIN
      | C-B*ACIN-C
      | C-B*ACIN-C-CARRYIN
      | C-B*ACIN-CARRYIN
      | C-B*ACIN-P
      | C-B*ACIN-P-CARRYIN
      | C-B*D
      | C-B*D-C
      | C-B*D-C-CARRYIN
      | C-B*D-CARRYIN
      | C-B*D-P
      | C-B*D-P-CARRYIN
      | C-BCIN*(A+D)
      | C-BCIN*(A+D)-C
      | C-BCIN*(A+D)-C-CARRYIN
      | C-BCIN*(A+D)-CARRYIN
      | C-BCIN*(A+D)-P
      | C-BCIN*(A+D)-P-CARRYIN
      | C-BCIN*(ACIN+D)
      | C-BCIN*(ACIN+D)-C
      | C-BCIN*(ACIN+D)-C-CARRYIN
      | C-BCIN*(ACIN+D)-CARRYIN
      | C-BCIN*(ACIN+D)-P
      | C-BCIN*(ACIN+D)-P-CARRYIN
      | C-BCIN*(D+A)
      | C-BCIN*(D+A)-C
      | C-BCIN*(D+A)-C-CARRYIN
      | C-BCIN*(D+A)-CARRYIN
      | C-BCIN*(D+A)-P
      | C-BCIN*(D+A)-P-CARRYIN
      | C-BCIN*(D+ACIN)
      | C-BCIN*(D+ACIN)-C
      | C-BCIN*(D+ACIN)-C-CARRYIN
      | C-BCIN*(D+ACIN)-CARRYIN
      | C-BCIN*(D+ACIN)-P
      | C-BCIN*(D+ACIN)-P-CARRYIN
      | C-BCIN*(D-A)
      | C-BCIN*(D-A)-C
      | C-BCIN*(D-A)-C-CARRYIN
      | C-BCIN*(D-A)-CARRYIN
      | C-BCIN*(D-A)-P
      | C-BCIN*(D-A)-P-CARRYIN
      | C-BCIN*(D-ACIN)
      | C-BCIN*(D-ACIN)-C
      | C-BCIN*(D-ACIN)-C-CARRYIN
      | C-BCIN*(D-ACIN)-CARRYIN
      | C-BCIN*(D-ACIN)-P
      | C-BCIN*(D-ACIN)-P-CARRYIN
      | C-BCIN*A
      | C-BCIN*A-C
      | C-BCIN*A-C-CARRYIN
      | C-BCIN*A-CARRYIN
      | C-BCIN*A-P
      | C-BCIN*A-P-CARRYIN
      | C-BCIN*ACIN
      | C-BCIN*ACIN-C
      | C-BCIN*ACIN-C-CARRYIN
      | C-BCIN*ACIN-CARRYIN
      | C-BCIN*ACIN-P
      | C-BCIN*ACIN-P-CARRYIN
      | C-BCIN*D
      | C-BCIN*D-C
      | C-BCIN*D-C-CARRYIN
      | C-BCIN*D-CARRYIN
      | C-BCIN*D-P
      | C-BCIN*D-P-CARRYIN
      | C-C
      | C-C-(A+D)
      | C-C-(A+D)*(A+D)
      | C-C-(A+D)*(A+D)-CARRYIN
      | C-C-(A+D)*B
      | C-C-(A+D)*B-CARRYIN
      | C-C-(A+D)*BCIN
      | C-C-(A+D)*BCIN-CARRYIN
      | C-C-(A+D)-CARRYIN
      | C-C-(ACIN+D)
      | C-C-(ACIN+D)*(ACIN+D)
      | C-C-(ACIN+D)*(ACIN+D)-CARRYIN
      | C-C-(ACIN+D)*B
      | C-C-(ACIN+D)*B-CARRYIN
      | C-C-(ACIN+D)*BCIN
      | C-C-(ACIN+D)*BCIN-CARRYIN
      | C-C-(ACIN+D)-CARRYIN
      | C-C-(D+A)
      | C-C-(D+A)*(D+A)
      | C-C-(D+A)*(D+A)-CARRYIN
      | C-C-(D+A)*B
      | C-C-(D+A)*B-CARRYIN
      | C-C-(D+A)*BCIN
      | C-C-(D+A)*BCIN-CARRYIN
      | C-C-(D+A)-CARRYIN
      | C-C-(D+ACIN)
      | C-C-(D+ACIN)*(D+ACIN)
      | C-C-(D+ACIN)*(D+ACIN)-CARRYIN
      | C-C-(D+ACIN)*B
      | C-C-(D+ACIN)*B-CARRYIN
      | C-C-(D+ACIN)*BCIN
      | C-C-(D+ACIN)*BCIN-CARRYIN
      | C-C-(D+ACIN)-CARRYIN
      | C-C-(D-A)
      | C-C-(D-A)*(D-A)
      | C-C-(D-A)*(D-A)-CARRYIN
      | C-C-(D-A)*B
      | C-C-(D-A)*B-CARRYIN
      | C-C-(D-A)*BCIN
      | C-C-(D-A)*BCIN-CARRYIN
      | C-C-(D-A)-CARRYIN
      | C-C-(D-ACIN)
      | C-C-(D-ACIN)*(D-ACIN)
      | C-C-(D-ACIN)*(D-ACIN)-CARRYIN
      | C-C-(D-ACIN)*B
      | C-C-(D-ACIN)*B-CARRYIN
      | C-C-(D-ACIN)*BCIN
      | C-C-(D-ACIN)*BCIN-CARRYIN
      | C-C-(D-ACIN)-CARRYIN
      | C-C-A
      | C-C-A*A
      | C-C-A*A-CARRYIN
      | C-C-A*B
      | C-C-A*B-CARRYIN
      | C-C-A*BCIN
      | C-C-A*BCIN-CARRYIN
      | C-C-A-CARRYIN
      | C-C-ACIN
      | C-C-ACIN*ACIN
      | C-C-ACIN*ACIN-CARRYIN
      | C-C-ACIN*B
      | C-C-ACIN*B-CARRYIN
      | C-C-ACIN*BCIN
      | C-C-ACIN*BCIN-CARRYIN
      | C-C-ACIN-CARRYIN
      | C-C-B*(A+D)
      | C-C-B*(A+D)-CARRYIN
      | C-C-B*(ACIN+D)
      | C-C-B*(ACIN+D)-CARRYIN
      | C-C-B*(D+A)
      | C-C-B*(D+A)-CARRYIN
      | C-C-B*(D+ACIN)
      | C-C-B*(D+ACIN)-CARRYIN
      | C-C-B*(D-A)
      | C-C-B*(D-A)-CARRYIN
      | C-C-B*(D-ACIN)
      | C-C-B*(D-ACIN)-CARRYIN
      | C-C-B*A
      | C-C-B*A-CARRYIN
      | C-C-B*ACIN
      | C-C-B*ACIN-CARRYIN
      | C-C-B*D
      | C-C-B*D-CARRYIN
      | C-C-BCIN*(A+D)
      | C-C-BCIN*(A+D)-CARRYIN
      | C-C-BCIN*(ACIN+D)
      | C-C-BCIN*(ACIN+D)-CARRYIN
      | C-C-BCIN*(D+A)
      | C-C-BCIN*(D+A)-CARRYIN
      | C-C-BCIN*(D+ACIN)
      | C-C-BCIN*(D+ACIN)-CARRYIN
      | C-C-BCIN*(D-A)
      | C-C-BCIN*(D-A)-CARRYIN
      | C-C-BCIN*(D-ACIN)
      | C-C-BCIN*(D-ACIN)-CARRYIN
      | C-C-BCIN*A
      | C-C-BCIN*A-CARRYIN
      | C-C-BCIN*ACIN
      | C-C-BCIN*ACIN-CARRYIN
      | C-C-BCIN*D
      | C-C-BCIN*D-CARRYIN
      | C-C-C
      | C-C-C-CARRYIN
      | C-C-C-CONCAT
      | C-C-C-CONCAT-CARRYIN
      | C-C-C-P
      | C-C-C-P-CARRYIN
      | C-C-CARRYCASCIN
      | C-C-CARRYIN
      | C-C-CONCAT
      | C-C-CONCAT-C
      | C-C-CONCAT-C-CARRYIN
      | C-C-CONCAT-CARRYIN
      | C-C-CONCAT-P
      | C-C-CONCAT-P-CARRYIN
      | C-C-D
      | C-C-D*B
      | C-C-D*B-CARRYIN
      | C-C-D*BCIN
      | C-C-D*BCIN-CARRYIN
      | C-C-D*D
      | C-C-D*D-CARRYIN
      | C-C-D-CARRYIN
      | C-C-P
      | C-C-P-C
      | C-C-P-C-CARRYIN
      | C-C-P-CARRYIN
      | C-C-P-CONCAT
      | C-C-P-CONCAT-CARRYIN
      | C-C-P-P
      | C-C-P-P-CARRYIN
      | C-CARRYCASCIN
      | C-CARRYIN
      | C-CONCAT
      | C-CONCAT-C
      | C-CONCAT-C-C
      | C-CONCAT-C-C-CARRYIN
      | C-CONCAT-C-CARRYIN
      | C-CONCAT-C-P
      | C-CONCAT-C-P-CARRYIN
      | C-CONCAT-CARRYCASCIN
      | C-CONCAT-CARRYIN
      | C-CONCAT-P
      | C-CONCAT-P-C
      | C-CONCAT-P-C-CARRYIN
      | C-CONCAT-P-CARRYIN
      | C-D
      | C-D*B
      | C-D*B-C
      | C-D*B-C-CARRYIN
      | C-D*B-CARRYIN
      | C-D*B-P
      | C-D*B-P-CARRYIN
      | C-D*BCIN
      | C-D*BCIN-C
      | C-D*BCIN-C-CARRYIN
      | C-D*BCIN-CARRYIN
      | C-D*BCIN-P
      | C-D*BCIN-P-CARRYIN
      | C-D*D
      | C-D*D-C
      | C-D*D-C-CARRYIN
      | C-D*D-CARRYIN
      | C-D*D-P
      | C-D*D-P-CARRYIN
      | C-D-C
      | C-D-C-CARRYIN
      | C-D-CARRYIN
      | C-D-P
      | C-D-P-CARRYIN
      | C-P
      | C-P-(A+D)
      | C-P-(A+D)*(A+D)
      | C-P-(A+D)*(A+D)-CARRYIN
      | C-P-(A+D)*B
      | C-P-(A+D)*B-CARRYIN
      | C-P-(A+D)*BCIN
      | C-P-(A+D)*BCIN-CARRYIN
      | C-P-(A+D)-CARRYIN
      | C-P-(ACIN+D)
      | C-P-(ACIN+D)*(ACIN+D)
      | C-P-(ACIN+D)*(ACIN+D)-CARRYIN
      | C-P-(ACIN+D)*B
      | C-P-(ACIN+D)*B-CARRYIN
      | C-P-(ACIN+D)*BCIN
      | C-P-(ACIN+D)*BCIN-CARRYIN